simulink model "ospfb_2x_8192c_8i_16o"  (R2021a)
settings: Solver=VariableStepAuto  StartTime=0.0  StopTime=2304  RelTol=1e-3
undefined symbols (not defined in the file or in its folder): pfb_coeff_gen_calc
libraries: xbsIndex_r4 x23880, casper_library_misc x142, casper_library_multipliers x88, casper_library_delays x68
(part 7 of 158)

== system ospfb_2x_8192c_8i_16o/pfb_fir/pol1_in1_coeffs10 ==
  din : Inport
  sync : Inport  Port=2
  Concat : xbsIndex_r4/Concat  num_inputs=8  dbl_ovrd=off  block_type=concat
      settings: block_version=9.1.01
  Concat{1..8} (8 alike) : xbsIndex_r4/Concat  num_inputs=2  dbl_ovrd=off  block_type=concat
  Constant{,3,12,17} (4 alike) : xbsIndex_r4/Constant  const=0  gui_display_data_type=Fixed-point  arith_type=Unsigned  n_bits=1  bin_pt=0  preci_type=Single  exp_width=8  frac_width=24  explicit_period=on  period=1  equ=P=C  opselect=C  inp2=PCIN>>17  opr=+  inp1=P  carry=CIN  dbl_ovrd=off  block_type=constant
  Constant{1,4,13,18} (4 alike) : xbsIndex_r4/Constant  const=1  gui_display_data_type=Fixed-point  arith_type=Unsigned  n_bits=1  bin_pt=0  preci_type=Single  exp_width=8  frac_width=24  explicit_period=on  period=1  equ=P=C  opselect=C  inp2=PCIN>>17  opr=+  inp1=P  carry=CIN  dbl_ovrd=off  block_type=constant
  Constant{2,6,8,10,14,16,20,22} (8 alike) : xbsIndex_r4/Constant  const=0  gui_display_data_type=Fixed-point  arith_type=Signed (2's comp)  n_bits=18  bin_pt=17  preci_type=Single  exp_width=8  frac_width=24  explicit_period=on  period=1  equ=P=C  opselect=C  inp2=PCIN>>17  opr=+  inp1=P  carry=CIN  dbl_ovrd=off  block_type=constant
  Constant{5,7,9,11,15,19,21,23} (8 alike) : xbsIndex_r4/Constant  const=0  gui_display_data_type=Boolean  arith_type=Boolean  n_bits=18  bin_pt=17  preci_type=Single  exp_width=8  frac_width=24  explicit_period=on  period=1  equ=P=C  opselect=C  inp2=PCIN>>17  opr=+  inp1=P  carry=CIN  dbl_ovrd=off  block_type=constant
  Counter : xbsIndex_r4/Counter  cnt_type=Free Running  cnt_to=Inf  operation=Up  start_count=0  cnt_by_val=1  arith_type=Unsigned  n_bits=9  bin_pt=0  load_pin=off  rst=on  en=off  explicit_period=on  period=1  use_behavioral_HDL=off  implementation=Fabric  use_rpm=on  dbl_ovrd=off  block_type=counter
      settings: xl_area=[0, 0, 0, 0, 0, 0, 0]  block_version=9.1.01
  Delay : xbsIndex_r4/Delay  rst=off  infoeditControl=Selection of Reset will increase slice count due to use of real FFs and instead of SRLs  en=off  latency=bram_latency+fan_latency  reg_retiming=off  dbl_ovrd=off  block_type=delay
      settings: xl_area=[0, 0, 0, 0, 0, 0, 0]  block_version=9.1.01
  Delay1 : xbsIndex_r4/Delay  rst=off  infoeditControl=Selection of Reset will increase slice count due to use of real FFs and instead of SRLs  en=off  latency=bram_latency+fan_latency  reg_retiming=off  dbl_ovrd=off  block_type=delay
      settings: xl_area=[0, 0, 0, 0, 0, 0, 0]  block_version=9.1.01
  Reinterpret{1..8} (8 alike) : xbsIndex_r4/Reinterpret  force_arith_type=on  arith_type=Unsigned  force_bin_pt=on  bin_pt=0  block_type=reinterpret
      Reinterpret1 settings: infoedit="Changes signal type without altering the binary representation.   You can changed the signal between signed and unsigned, and relocate the binary point.<P><P>Hardware notes: In hardware this block costs nothing.<P><P>Example:  Suppose the input is 6 bits wide, signed, with 2 fractional bits, and the output is forced to unsigned with 0 fractional bits.  Then an input of -2.0 (1110.00 in binary 2's complement) becomes an output of 56 (111000 in binary)."  block_version=9.1.01
      Reinterpret2 settings: infoedit="Changes signal type without altering the binary representation.   You can changed the signal between signed and unsigned, and relocate the binary point.<P><P>Hardware notes: In hardware this block costs nothing.<P><P>Example:  Suppose the input is 6 bits wide, signed, with 2 fractional bits, and the output is forced to unsigned with 0 fractional bits.  Then an input of -2.0 (1110.00 in binary 2's complement) becomes an output of 56 (111000 in binary)."  block_version=9.1.01
  c0 : xbsIndex_r4/Dual Port RAM  depth=1024  initVector=[pfb_coeff_gen_calc(12, 8,'dpss',n_inputs, nput,1,1,false), pfb_coeff_gen_calc(12, 8,'dpss',n_inputs, nput,1,2,false)]  distributed_mem=Block RAM  init_a=0  init_b=0  rst_a=off  rst_b=off  en_a=off  en_b=off  latency=1  write_mode_A=Read After Write  write_mode_B=Read After Write  optimize=Area  dbl_ovrd=off  simulation_type=fast_sim  block_type=dpram
  c1 : xbsIndex_r4/Dual Port RAM  depth=1024  initVector=[pfb_coeff_gen_calc(12, 8,'dpss',n_inputs, nput,1,3,false), pfb_coeff_gen_calc(12, 8,'dpss',n_inputs, nput,1,4,false)]  distributed_mem=Block RAM  init_a=0  init_b=0  rst_a=off  rst_b=off  en_a=off  en_b=off  latency=1  write_mode_A=Read After Write  write_mode_B=Read After Write  optimize=Area  dbl_ovrd=off  simulation_type=fast_sim  block_type=dpram
  c2 : xbsIndex_r4/Dual Port RAM  depth=1024  initVector=[pfb_coeff_gen_calc(12, 8,'dpss',n_inputs, nput,1,5,false), pfb_coeff_gen_calc(12, 8,'dpss',n_inputs, nput,1,6,false)]  distributed_mem=Block RAM  init_a=0  init_b=0  rst_a=off  rst_b=off  en_a=off  en_b=off  latency=1  write_mode_A=Read After Write  write_mode_B=Read After Write  optimize=Area  dbl_ovrd=off  simulation_type=fast_sim  block_type=dpram
  c3 : xbsIndex_r4/Dual Port RAM  depth=1024  initVector=[pfb_coeff_gen_calc(12, 8,'dpss',n_inputs, nput,1,7,false), pfb_coeff_gen_calc(12, 8,'dpss',n_inputs, nput,1,8,false)]  distributed_mem=Block RAM  init_a=0  init_b=0  rst_a=off  rst_b=off  en_a=off  en_b=off  latency=1  write_mode_A=Read After Write  write_mode_B=Read After Write  optimize=Area  dbl_ovrd=off  simulation_type=fast_sim  block_type=dpram
  fan_delay{1..8} (8 alike) : xbsIndex_r4/Delay  rst=off  infoeditControl=Selection of Reset will increase slice count due to use of real FFs and instead of SRLs  en=off  latency=fan_latency  reg_retiming=off  dbl_ovrd=off  block_type=delay
      fan_delay1 settings: block_version=11.4
      fan_delay2 settings: block_version=11.4
  dout : Outport  VectorParamsAs1DForOutWhenUnconnected=off
  sync_out : Outport  Port=2  VectorParamsAs1DForOutWhenUnconnected=off
  coeff : Outport  Port=3  VectorParamsAs1DForOutWhenUnconnected=off
  wires:
  din/1 -> Delay1/1
  Delay1/1 -> dout/1
  sync/1 -> Counter/1, Delay/1
  Delay/1 -> sync_out/1
  Concat/1 -> coeff/1
  Counter/1 -> fan_delay1/1, fan_delay2/1, fan_delay3/1, fan_delay4/1, fan_delay5/1, fan_delay6/1, fan_delay7/1, fan_delay8/1
  fan_delay1/1 -> Concat1/2
  Reinterpret1/1 -> Concat/1
  fan_delay2/1 -> Concat2/2
  Reinterpret2/1 -> Concat/2
  Reinterpret3/1 -> Concat/3
  Reinterpret4/1 -> Concat/4
  Reinterpret5/1 -> Concat/5
  Reinterpret6/1 -> Concat/6
  Reinterpret7/1 -> Concat/7
  Reinterpret8/1 -> Concat/8
  Constant/1 -> Concat1/1
  Constant1/1 -> Concat2/1
  Concat1/1 -> c0/1
  Constant2/1 -> c0/2
  Constant5/1 -> c0/3
  Constant7/1 -> c0/6
  Constant6/1 -> c0/5
  Concat2/1 -> c0/4
  c0/2 -> Reinterpret2/1
  c0/1 -> Reinterpret1/1
  c1/2 -> Reinterpret4/1
  Concat4/1 -> c1/4
  Constant10/1 -> c1/5
  Constant11/1 -> c1/6
  Constant9/1 -> c1/3
  Constant8/1 -> c1/2
  Concat3/1 -> c1/1
  Constant4/1 -> Concat4/1
  Constant3/1 -> Concat3/1
  fan_delay4/1 -> Concat4/2
  fan_delay3/1 -> Concat3/2
  fan_delay7/1 -> Concat7/2
  fan_delay8/1 -> Concat8/2
  Constant17/1 -> Concat7/1
  Constant18/1 -> Concat8/1
  Concat7/1 -> c3/1
  Constant22/1 -> c3/2
  Constant23/1 -> c3/3
  Constant15/1 -> c3/6
  Constant14/1 -> c3/5
  Concat8/1 -> c3/4
  Concat6/1 -> c2/4
  Constant20/1 -> c2/5
  Constant21/1 -> c2/6
  Constant19/1 -> c2/3
  Constant16/1 -> c2/2
  Concat5/1 -> c2/1
  Constant13/1 -> Concat6/1
  Constant12/1 -> Concat5/1
  fan_delay6/1 -> Concat6/2
  fan_delay5/1 -> Concat5/2
  c1/1 -> Reinterpret3/1
  c2/1 -> Reinterpret5/1
  c2/2 -> Reinterpret6/1
  c3/1 -> Reinterpret7/1
  c3/2 -> Reinterpret8/1

== system ospfb_2x_8192c_8i_16o/pfb_fir/pol1_in1_coeffs11 ==
  din : Inport
  sync : Inport  Port=2
  Concat : xbsIndex_r4/Concat  num_inputs=8  dbl_ovrd=off  block_type=concat
      settings: block_version=9.1.01
  Concat{1..8} (8 alike) : xbsIndex_r4/Concat  num_inputs=2  dbl_ovrd=off  block_type=concat
  Constant{,3,12,17} (4 alike) : xbsIndex_r4/Constant  const=0  gui_display_data_type=Fixed-point  arith_type=Unsigned  n_bits=1  bin_pt=0  preci_type=Single  exp_width=8  frac_width=24  explicit_period=on  period=1  equ=P=C  opselect=C  inp2=PCIN>>17  opr=+  inp1=P  carry=CIN  dbl_ovrd=off  block_type=constant
  Constant{1,4,13,18} (4 alike) : xbsIndex_r4/Constant  const=1  gui_display_data_type=Fixed-point  arith_type=Unsigned  n_bits=1  bin_pt=0  preci_type=Single  exp_width=8  frac_width=24  explicit_period=on  period=1  equ=P=C  opselect=C  inp2=PCIN>>17  opr=+  inp1=P  carry=CIN  dbl_ovrd=off  block_type=constant
  Constant{2,6,8,10,14,16,20,22} (8 alike) : xbsIndex_r4/Constant  const=0  gui_display_data_type=Fixed-point  arith_type=Signed (2's comp)  n_bits=18  bin_pt=17  preci_type=Single  exp_width=8  frac_width=24  explicit_period=on  period=1  equ=P=C  opselect=C  inp2=PCIN>>17  opr=+  inp1=P  carry=CIN  dbl_ovrd=off  block_type=constant
  Constant{5,7,9,11,15,19,21,23} (8 alike) : xbsIndex_r4/Constant  const=0  gui_display_data_type=Boolean  arith_type=Boolean  n_bits=18  bin_pt=17  preci_type=Single  exp_width=8  frac_width=24  explicit_period=on  period=1  equ=P=C  opselect=C  inp2=PCIN>>17  opr=+  inp1=P  carry=CIN  dbl_ovrd=off  block_type=constant
  Counter : xbsIndex_r4/Counter  cnt_type=Free Running  cnt_to=Inf  operation=Up  start_count=0  cnt_by_val=1  arith_type=Unsigned  n_bits=9  bin_pt=0  load_pin=off  rst=on  en=off  explicit_period=on  period=1  use_behavioral_HDL=off  implementation=Fabric  use_rpm=on  dbl_ovrd=off  block_type=counter
      settings: xl_area=[0, 0, 0, 0, 0, 0, 0]  block_version=9.1.01
  Delay : xbsIndex_r4/Delay  rst=off  infoeditControl=Selection of Reset will increase slice count due to use of real FFs and instead of SRLs  en=off  latency=bram_latency+fan_latency  reg_retiming=off  dbl_ovrd=off  block_type=delay
      settings: xl_area=[0, 0, 0, 0, 0, 0, 0]  block_version=9.1.01
  Delay1 : xbsIndex_r4/Delay  rst=off  infoeditControl=Selection of Reset will increase slice count due to use of real FFs and instead of SRLs  en=off  latency=bram_latency+fan_latency  reg_retiming=off  dbl_ovrd=off  block_type=delay
      settings: xl_area=[0, 0, 0, 0, 0, 0, 0]  block_version=9.1.01
  Reinterpret{1..8} (8 alike) : xbsIndex_r4/Reinterpret  force_arith_type=on  arith_type=Unsigned  force_bin_pt=on  bin_pt=0  block_type=reinterpret
      Reinterpret1 settings: infoedit="Changes signal type without altering the binary representation.   You can changed the signal between signed and unsigned, and relocate the binary point.<P><P>Hardware notes: In hardware this block costs nothing.<P><P>Example:  Suppose the input is 6 bits wide, signed, with 2 fractional bits, and the output is forced to unsigned with 0 fractional bits.  Then an input of -2.0 (1110.00 in binary 2's complement) becomes an output of 56 (111000 in binary)."  block_version=9.1.01
      Reinterpret2 settings: infoedit="Changes signal type without altering the binary representation.   You can changed the signal between signed and unsigned, and relocate the binary point.<P><P>Hardware notes: In hardware this block costs nothing.<P><P>Example:  Suppose the input is 6 bits wide, signed, with 2 fractional bits, and the output is forced to unsigned with 0 fractional bits.  Then an input of -2.0 (1110.00 in binary 2's complement) becomes an output of 56 (111000 in binary)."  block_version=9.1.01
  c0 : xbsIndex_r4/Dual Port RAM  depth=1024  initVector=[pfb_coeff_gen_calc(12, 8,'dpss',n_inputs, nput,1,1,false), pfb_coeff_gen_calc(12, 8,'dpss',n_inputs, nput,1,2,false)]  distributed_mem=Block RAM  init_a=0  init_b=0  rst_a=off  rst_b=off  en_a=off  en_b=off  latency=1  write_mode_A=Read After Write  write_mode_B=Read After Write  optimize=Area  dbl_ovrd=off  simulation_type=fast_sim  block_type=dpram
  c1 : xbsIndex_r4/Dual Port RAM  depth=1024  initVector=[pfb_coeff_gen_calc(12, 8,'dpss',n_inputs, nput,1,3,false), pfb_coeff_gen_calc(12, 8,'dpss',n_inputs, nput,1,4,false)]  distributed_mem=Block RAM  init_a=0  init_b=0  rst_a=off  rst_b=off  en_a=off  en_b=off  latency=1  write_mode_A=Read After Write  write_mode_B=Read After Write  optimize=Area  dbl_ovrd=off  simulation_type=fast_sim  block_type=dpram
  c2 : xbsIndex_r4/Dual Port RAM  depth=1024  initVector=[pfb_coeff_gen_calc(12, 8,'dpss',n_inputs, nput,1,5,false), pfb_coeff_gen_calc(12, 8,'dpss',n_inputs, nput,1,6,false)]  distributed_mem=Block RAM  init_a=0  init_b=0  rst_a=off  rst_b=off  en_a=off  en_b=off  latency=1  write_mode_A=Read After Write  write_mode_B=Read After Write  optimize=Area  dbl_ovrd=off  simulation_type=fast_sim  block_type=dpram
  c3 : xbsIndex_r4/Dual Port RAM  depth=1024  initVector=[pfb_coeff_gen_calc(12, 8,'dpss',n_inputs, nput,1,7,false), pfb_coeff_gen_calc(12, 8,'dpss',n_inputs, nput,1,8,false)]  distributed_mem=Block RAM  init_a=0  init_b=0  rst_a=off  rst_b=off  en_a=off  en_b=off  latency=1  write_mode_A=Read After Write  write_mode_B=Read After Write  optimize=Area  dbl_ovrd=off  simulation_type=fast_sim  block_type=dpram
  fan_delay{1..8} (8 alike) : xbsIndex_r4/Delay  rst=off  infoeditControl=Selection of Reset will increase slice count due to use of real FFs and instead of SRLs  en=off  latency=fan_latency  reg_retiming=off  dbl_ovrd=off  block_type=delay
      fan_delay1 settings: block_version=11.4
      fan_delay2 settings: block_version=11.4
  dout : Outport  VectorParamsAs1DForOutWhenUnconnected=off
  sync_out : Outport  Port=2  VectorParamsAs1DForOutWhenUnconnected=off
  coeff : Outport  Port=3  VectorParamsAs1DForOutWhenUnconnected=off
  wires:
  c3/2 -> Reinterpret8/1
  c3/1 -> Reinterpret7/1
  c2/2 -> Reinterpret6/1
  c2/1 -> Reinterpret5/1
  c1/1 -> Reinterpret3/1
  fan_delay5/1 -> Concat5/2
  fan_delay6/1 -> Concat6/2
  Constant12/1 -> Concat5/1
  Constant13/1 -> Concat6/1
  Concat5/1 -> c2/1
  Constant16/1 -> c2/2
  Constant19/1 -> c2/3
  Constant21/1 -> c2/6
  Constant20/1 -> c2/5
  Concat6/1 -> c2/4
  Concat8/1 -> c3/4
  Constant14/1 -> c3/5
  Constant15/1 -> c3/6
  Constant23/1 -> c3/3
  Constant22/1 -> c3/2
  Concat7/1 -> c3/1
  Constant18/1 -> Concat8/1
  Constant17/1 -> Concat7/1
  fan_delay8/1 -> Concat8/2
  fan_delay7/1 -> Concat7/2
  fan_delay3/1 -> Concat3/2
  fan_delay4/1 -> Concat4/2
  Constant3/1 -> Concat3/1
  Constant4/1 -> Concat4/1
  Concat3/1 -> c1/1
  Constant8/1 -> c1/2
  Constant9/1 -> c1/3
  Constant11/1 -> c1/6
  Constant10/1 -> c1/5
  Concat4/1 -> c1/4
  c1/2 -> Reinterpret4/1
  c0/1 -> Reinterpret1/1
  c0/2 -> Reinterpret2/1
  Concat2/1 -> c0/4
  Constant6/1 -> c0/5
  Constant7/1 -> c0/6
  Constant5/1 -> c0/3
  Constant2/1 -> c0/2
  Concat1/1 -> c0/1
  Constant1/1 -> Concat2/1
  Constant/1 -> Concat1/1
  Reinterpret8/1 -> Concat/8
  Reinterpret7/1 -> Concat/7
  Reinterpret6/1 -> Concat/6
  Reinterpret5/1 -> Concat/5
  Reinterpret4/1 -> Concat/4
  Reinterpret3/1 -> Concat/3
  Reinterpret2/1 -> Concat/2
  fan_delay2/1 -> Concat2/2
  Reinterpret1/1 -> Concat/1
  fan_delay1/1 -> Concat1/2
  Counter/1 -> fan_delay8/1, fan_delay7/1, fan_delay6/1, fan_delay5/1, fan_delay4/1, fan_delay3/1, fan_delay2/1, fan_delay1/1
  Concat/1 -> coeff/1
  Delay/1 -> sync_out/1
  sync/1 -> Delay/1, Counter/1
  Delay1/1 -> dout/1
  din/1 -> Delay1/1

== system ospfb_2x_8192c_8i_16o/pfb_fir/pol1_in1_coeffs12 ==
  din : Inport
  sync : Inport  Port=2
  Concat : xbsIndex_r4/Concat  num_inputs=8  dbl_ovrd=off  block_type=concat
      settings: block_version=9.1.01
  Concat{1..8} (8 alike) : xbsIndex_r4/Concat  num_inputs=2  dbl_ovrd=off  block_type=concat
  Constant{,3,12,17} (4 alike) : xbsIndex_r4/Constant  const=0  gui_display_data_type=Fixed-point  arith_type=Unsigned  n_bits=1  bin_pt=0  preci_type=Single  exp_width=8  frac_width=24  explicit_period=on  period=1  equ=P=C  opselect=C  inp2=PCIN>>17  opr=+  inp1=P  carry=CIN  dbl_ovrd=off  block_type=constant
  Constant{1,4,13,18} (4 alike) : xbsIndex_r4/Constant  const=1  gui_display_data_type=Fixed-point  arith_type=Unsigned  n_bits=1  bin_pt=0  preci_type=Single  exp_width=8  frac_width=24  explicit_period=on  period=1  equ=P=C  opselect=C  inp2=PCIN>>17  opr=+  inp1=P  carry=CIN  dbl_ovrd=off  block_type=constant
  Constant{2,6,8,10,14,16,20,22} (8 alike) : xbsIndex_r4/Constant  const=0  gui_display_data_type=Fixed-point  arith_type=Signed (2's comp)  n_bits=18  bin_pt=17  preci_type=Single  exp_width=8  frac_width=24  explicit_period=on  period=1  equ=P=C  opselect=C  inp2=PCIN>>17  opr=+  inp1=P  carry=CIN  dbl_ovrd=off  block_type=constant
  Constant{5,7,9,11,15,19,21,23} (8 alike) : xbsIndex_r4/Constant  const=0  gui_display_data_type=Boolean  arith_type=Boolean  n_bits=18  bin_pt=17  preci_type=Single  exp_width=8  frac_width=24  explicit_period=on  period=1  equ=P=C  opselect=C  inp2=PCIN>>17  opr=+  inp1=P  carry=CIN  dbl_ovrd=off  block_type=constant
  Counter : xbsIndex_r4/Counter  cnt_type=Free Running  cnt_to=Inf  operation=Up  start_count=0  cnt_by_val=1  arith_type=Unsigned  n_bits=9  bin_pt=0  load_pin=off  rst=on  en=off  explicit_period=on  period=1  use_behavioral_HDL=off  implementation=Fabric  use_rpm=on  dbl_ovrd=off  block_type=counter
      settings: xl_area=[0, 0, 0, 0, 0, 0, 0]  block_version=9.1.01
  Delay : xbsIndex_r4/Delay  rst=off  infoeditControl=Selection of Reset will increase slice count due to use of real FFs and instead of SRLs  en=off  latency=bram_latency+fan_latency  reg_retiming=off  dbl_ovrd=off  block_type=delay
      settings: xl_area=[0, 0, 0, 0, 0, 0, 0]  block_version=9.1.01
  Delay1 : xbsIndex_r4/Delay  rst=off  infoeditControl=Selection of Reset will increase slice count due to use of real FFs and instead of SRLs  en=off  latency=bram_latency+fan_latency  reg_retiming=off  dbl_ovrd=off  block_type=delay
      settings: xl_area=[0, 0, 0, 0, 0, 0, 0]  block_version=9.1.01
  Reinterpret{1..8} (8 alike) : xbsIndex_r4/Reinterpret  force_arith_type=on  arith_type=Unsigned  force_bin_pt=on  bin_pt=0  block_type=reinterpret
      Reinterpret1 settings: infoedit="Changes signal type without altering the binary representation.   You can changed the signal between signed and unsigned, and relocate the binary point.<P><P>Hardware notes: In hardware this block costs nothing.<P><P>Example:  Suppose the input is 6 bits wide, signed, with 2 fractional bits, and the output is forced to unsigned with 0 fractional bits.  Then an input of -2.0 (1110.00 in binary 2's complement) becomes an output of 56 (111000 in binary)."  block_version=9.1.01
      Reinterpret2 settings: infoedit="Changes signal type without altering the binary representation.   You can changed the signal between signed and unsigned, and relocate the binary point.<P><P>Hardware notes: In hardware this block costs nothing.<P><P>Example:  Suppose the input is 6 bits wide, signed, with 2 fractional bits, and the output is forced to unsigned with 0 fractional bits.  Then an input of -2.0 (1110.00 in binary 2's complement) becomes an output of 56 (111000 in binary)."  block_version=9.1.01
  c0 : xbsIndex_r4/Dual Port RAM  depth=1024  initVector=[pfb_coeff_gen_calc(12, 8,'dpss',n_inputs, nput,1,1,false), pfb_coeff_gen_calc(12, 8,'dpss',n_inputs, nput,1,2,false)]  distributed_mem=Block RAM  init_a=0  init_b=0  rst_a=off  rst_b=off  en_a=off  en_b=off  latency=1  write_mode_A=Read After Write  write_mode_B=Read After Write  optimize=Area  dbl_ovrd=off  simulation_type=fast_sim  block_type=dpram
  c1 : xbsIndex_r4/Dual Port RAM  depth=1024  initVector=[pfb_coeff_gen_calc(12, 8,'dpss',n_inputs, nput,1,3,false), pfb_coeff_gen_calc(12, 8,'dpss',n_inputs, nput,1,4,false)]  distributed_mem=Block RAM  init_a=0  init_b=0  rst_a=off  rst_b=off  en_a=off  en_b=off  latency=1  write_mode_A=Read After Write  write_mode_B=Read After Write  optimize=Area  dbl_ovrd=off  simulation_type=fast_sim  block_type=dpram
  c2 : xbsIndex_r4/Dual Port RAM  depth=1024  initVector=[pfb_coeff_gen_calc(12, 8,'dpss',n_inputs, nput,1,5,false), pfb_coeff_gen_calc(12, 8,'dpss',n_inputs, nput,1,6,false)]  distributed_mem=Block RAM  init_a=0  init_b=0  rst_a=off  rst_b=off  en_a=off  en_b=off  latency=1  write_mode_A=Read After Write  write_mode_B=Read After Write  optimize=Area  dbl_ovrd=off  simulation_type=fast_sim  block_type=dpram
  c3 : xbsIndex_r4/Dual Port RAM  depth=1024  initVector=[pfb_coeff_gen_calc(12, 8,'dpss',n_inputs, nput,1,7,false), pfb_coeff_gen_calc(12, 8,'dpss',n_inputs, nput,1,8,false)]  distributed_mem=Block RAM  init_a=0  init_b=0  rst_a=off  rst_b=off  en_a=off  en_b=off  latency=1  write_mode_A=Read After Write  write_mode_B=Read After Write  optimize=Area  dbl_ovrd=off  simulation_type=fast_sim  block_type=dpram
  fan_delay{1..8} (8 alike) : xbsIndex_r4/Delay  rst=off  infoeditControl=Selection of Reset will increase slice count due to use of real FFs and instead of SRLs  en=off  latency=fan_latency  reg_retiming=off  dbl_ovrd=off  block_type=delay
      fan_delay1 settings: block_version=11.4
      fan_delay2 settings: block_version=11.4
  dout : Outport  VectorParamsAs1DForOutWhenUnconnected=off
  sync_out : Outport  Port=2  VectorParamsAs1DForOutWhenUnconnected=off
  coeff : Outport  Port=3  VectorParamsAs1DForOutWhenUnconnected=off
  wires:
  din/1 -> Delay1/1
  Delay1/1 -> dout/1
  sync/1 -> Counter/1, Delay/1
  Delay/1 -> sync_out/1
  Concat/1 -> coeff/1
  Counter/1 -> fan_delay1/1, fan_delay2/1, fan_delay3/1, fan_delay4/1, fan_delay5/1, fan_delay6/1, fan_delay7/1, fan_delay8/1
  fan_delay1/1 -> Concat1/2
  Reinterpret1/1 -> Concat/1
  fan_delay2/1 -> Concat2/2
  Reinterpret2/1 -> Concat/2
  Reinterpret3/1 -> Concat/3
  Reinterpret4/1 -> Concat/4
  Reinterpret5/1 -> Concat/5
  Reinterpret6/1 -> Concat/6
  Reinterpret7/1 -> Concat/7
  Reinterpret8/1 -> Concat/8
  Constant/1 -> Concat1/1
  Constant1/1 -> Concat2/1
  Concat1/1 -> c0/1
  Constant2/1 -> c0/2
  Constant5/1 -> c0/3
  Constant7/1 -> c0/6
  Constant6/1 -> c0/5
  Concat2/1 -> c0/4
  c0/2 -> Reinterpret2/1
  c0/1 -> Reinterpret1/1
  c1/2 -> Reinterpret4/1
  Concat4/1 -> c1/4
  Constant10/1 -> c1/5
  Constant11/1 -> c1/6
  Constant9/1 -> c1/3
  Constant8/1 -> c1/2
  Concat3/1 -> c1/1
  Constant4/1 -> Concat4/1
  Constant3/1 -> Concat3/1
  fan_delay4/1 -> Concat4/2
  fan_delay3/1 -> Concat3/2
  fan_delay7/1 -> Concat7/2
  fan_delay8/1 -> Concat8/2
  Constant17/1 -> Concat7/1
  Constant18/1 -> Concat8/1
  Concat7/1 -> c3/1
  Constant22/1 -> c3/2
  Constant23/1 -> c3/3
  Constant15/1 -> c3/6
  Constant14/1 -> c3/5
  Concat8/1 -> c3/4
  Concat6/1 -> c2/4
  Constant20/1 -> c2/5
  Constant21/1 -> c2/6
  Constant19/1 -> c2/3
  Constant16/1 -> c2/2
  Concat5/1 -> c2/1
  Constant13/1 -> Concat6/1
  Constant12/1 -> Concat5/1
  fan_delay6/1 -> Concat6/2
  fan_delay5/1 -> Concat5/2
  c1/1 -> Reinterpret3/1
  c2/1 -> Reinterpret5/1
  c2/2 -> Reinterpret6/1
  c3/1 -> Reinterpret7/1
  c3/2 -> Reinterpret8/1

== system ospfb_2x_8192c_8i_16o/pfb_fir/pol1_in1_coeffs13 ==
  din : Inport
  sync : Inport  Port=2
  Concat : xbsIndex_r4/Concat  num_inputs=8  dbl_ovrd=off  block_type=concat
      settings: block_version=9.1.01
  Concat{1..8} (8 alike) : xbsIndex_r4/Concat  num_inputs=2  dbl_ovrd=off  block_type=concat
  Constant{,3,12,17} (4 alike) : xbsIndex_r4/Constant  const=0  gui_display_data_type=Fixed-point  arith_type=Unsigned  n_bits=1  bin_pt=0  preci_type=Single  exp_width=8  frac_width=24  explicit_period=on  period=1  equ=P=C  opselect=C  inp2=PCIN>>17  opr=+  inp1=P  carry=CIN  dbl_ovrd=off  block_type=constant
  Constant{1,4,13,18} (4 alike) : xbsIndex_r4/Constant  const=1  gui_display_data_type=Fixed-point  arith_type=Unsigned  n_bits=1  bin_pt=0  preci_type=Single  exp_width=8  frac_width=24  explicit_period=on  period=1  equ=P=C  opselect=C  inp2=PCIN>>17  opr=+  inp1=P  carry=CIN  dbl_ovrd=off  block_type=constant
  Constant{2,6,8,10,14,16,20,22} (8 alike) : xbsIndex_r4/Constant  const=0  gui_display_data_type=Fixed-point  arith_type=Signed (2's comp)  n_bits=18  bin_pt=17  preci_type=Single  exp_width=8  frac_width=24  explicit_period=on  period=1  equ=P=C  opselect=C  inp2=PCIN>>17  opr=+  inp1=P  carry=CIN  dbl_ovrd=off  block_type=constant
  Constant{5,7,9,11,15,19,21,23} (8 alike) : xbsIndex_r4/Constant  const=0  gui_display_data_type=Boolean  arith_type=Boolean  n_bits=18  bin_pt=17  preci_type=Single  exp_width=8  frac_width=24  explicit_period=on  period=1  equ=P=C  opselect=C  inp2=PCIN>>17  opr=+  inp1=P  carry=CIN  dbl_ovrd=off  block_type=constant
  Counter : xbsIndex_r4/Counter  cnt_type=Free Running  cnt_to=Inf  operation=Up  start_count=0  cnt_by_val=1  arith_type=Unsigned  n_bits=9  bin_pt=0  load_pin=off  rst=on  en=off  explicit_period=on  period=1  use_behavioral_HDL=off  implementation=Fabric  use_rpm=on  dbl_ovrd=off  block_type=counter
      settings: xl_area=[0, 0, 0, 0, 0, 0, 0]  block_version=9.1.01
  Delay : xbsIndex_r4/Delay  rst=off  infoeditControl=Selection of Reset will increase slice count due to use of real FFs and instead of SRLs  en=off  latency=bram_latency+fan_latency  reg_retiming=off  dbl_ovrd=off  block_type=delay
      settings: xl_area=[0, 0, 0, 0, 0, 0, 0]  block_version=9.1.01
  Delay1 : xbsIndex_r4/Delay  rst=off  infoeditControl=Selection of Reset will increase slice count due to use of real FFs and instead of SRLs  en=off  latency=bram_latency+fan_latency  reg_retiming=off  dbl_ovrd=off  block_type=delay
      settings: xl_area=[0, 0, 0, 0, 0, 0, 0]  block_version=9.1.01
  Reinterpret{1..8} (8 alike) : xbsIndex_r4/Reinterpret  force_arith_type=on  arith_type=Unsigned  force_bin_pt=on  bin_pt=0  block_type=reinterpret
      Reinterpret1 settings: infoedit="Changes signal type without altering the binary representation.   You can changed the signal between signed and unsigned, and relocate the binary point.<P><P>Hardware notes: In hardware this block costs nothing.<P><P>Example:  Suppose the input is 6 bits wide, signed, with 2 fractional bits, and the output is forced to unsigned with 0 fractional bits.  Then an input of -2.0 (1110.00 in binary 2's complement) becomes an output of 56 (111000 in binary)."  block_version=9.1.01
      Reinterpret2 settings: infoedit="Changes signal type without altering the binary representation.   You can changed the signal between signed and unsigned, and relocate the binary point.<P><P>Hardware notes: In hardware this block costs nothing.<P><P>Example:  Suppose the input is 6 bits wide, signed, with 2 fractional bits, and the output is forced to unsigned with 0 fractional bits.  Then an input of -2.0 (1110.00 in binary 2's complement) becomes an output of 56 (111000 in binary)."  block_version=9.1.01
  c0 : xbsIndex_r4/Dual Port RAM  depth=1024  initVector=[pfb_coeff_gen_calc(12, 8,'dpss',n_inputs, nput,1,1,false), pfb_coeff_gen_calc(12, 8,'dpss',n_inputs, nput,1,2,false)]  distributed_mem=Block RAM  init_a=0  init_b=0  rst_a=off  rst_b=off  en_a=off  en_b=off  latency=1  write_mode_A=Read After Write  write_mode_B=Read After Write  optimize=Area  dbl_ovrd=off  simulation_type=fast_sim  block_type=dpram
  c1 : xbsIndex_r4/Dual Port RAM  depth=1024  initVector=[pfb_coeff_gen_calc(12, 8,'dpss',n_inputs, nput,1,3,false), pfb_coeff_gen_calc(12, 8,'dpss',n_inputs, nput,1,4,false)]  distributed_mem=Block RAM  init_a=0  init_b=0  rst_a=off  rst_b=off  en_a=off  en_b=off  latency=1  write_mode_A=Read After Write  write_mode_B=Read After Write  optimize=Area  dbl_ovrd=off  simulation_type=fast_sim  block_type=dpram
  c2 : xbsIndex_r4/Dual Port RAM  depth=1024  initVector=[pfb_coeff_gen_calc(12, 8,'dpss',n_inputs, nput,1,5,false), pfb_coeff_gen_calc(12, 8,'dpss',n_inputs, nput,1,6,false)]  distributed_mem=Block RAM  init_a=0  init_b=0  rst_a=off  rst_b=off  en_a=off  en_b=off  latency=1  write_mode_A=Read After Write  write_mode_B=Read After Write  optimize=Area  dbl_ovrd=off  simulation_type=fast_sim  block_type=dpram
  c3 : xbsIndex_r4/Dual Port RAM  depth=1024  initVector=[pfb_coeff_gen_calc(12, 8,'dpss',n_inputs, nput,1,7,false), pfb_coeff_gen_calc(12, 8,'dpss',n_inputs, nput,1,8,false)]  distributed_mem=Block RAM  init_a=0  init_b=0  rst_a=off  rst_b=off  en_a=off  en_b=off  latency=1  write_mode_A=Read After Write  write_mode_B=Read After Write  optimize=Area  dbl_ovrd=off  simulation_type=fast_sim  block_type=dpram
  fan_delay{1..8} (8 alike) : xbsIndex_r4/Delay  rst=off  infoeditControl=Selection of Reset will increase slice count due to use of real FFs and instead of SRLs  en=off  latency=fan_latency  reg_retiming=off  dbl_ovrd=off  block_type=delay
      fan_delay1 settings: block_version=11.4
      fan_delay2 settings: block_version=11.4
  dout : Outport  VectorParamsAs1DForOutWhenUnconnected=off
  sync_out : Outport  Port=2  VectorParamsAs1DForOutWhenUnconnected=off
  coeff : Outport  Port=3  VectorParamsAs1DForOutWhenUnconnected=off
  wires:
  c3/2 -> Reinterpret8/1
  c3/1 -> Reinterpret7/1
  c2/2 -> Reinterpret6/1
  c2/1 -> Reinterpret5/1
  c1/1 -> Reinterpret3/1
  fan_delay5/1 -> Concat5/2
  fan_delay6/1 -> Concat6/2
  Constant12/1 -> Concat5/1
  Constant13/1 -> Concat6/1
  Concat5/1 -> c2/1
  Constant16/1 -> c2/2
  Constant19/1 -> c2/3
  Constant21/1 -> c2/6
  Constant20/1 -> c2/5
  Concat6/1 -> c2/4
  Concat8/1 -> c3/4
  Constant14/1 -> c3/5
  Constant15/1 -> c3/6
  Constant23/1 -> c3/3
  Constant22/1 -> c3/2
  Concat7/1 -> c3/1
  Constant18/1 -> Concat8/1
  Constant17/1 -> Concat7/1
  fan_delay8/1 -> Concat8/2
  fan_delay7/1 -> Concat7/2
  fan_delay3/1 -> Concat3/2
  fan_delay4/1 -> Concat4/2
  Constant3/1 -> Concat3/1
  Constant4/1 -> Concat4/1
  Concat3/1 -> c1/1
  Constant8/1 -> c1/2
  Constant9/1 -> c1/3
  Constant11/1 -> c1/6
  Constant10/1 -> c1/5
  Concat4/1 -> c1/4
  c1/2 -> Reinterpret4/1
  c0/1 -> Reinterpret1/1
  c0/2 -> Reinterpret2/1
  Concat2/1 -> c0/4
  Constant6/1 -> c0/5
  Constant7/1 -> c0/6
  Constant5/1 -> c0/3
  Constant2/1 -> c0/2
  Concat1/1 -> c0/1
  Constant1/1 -> Concat2/1
  Constant/1 -> Concat1/1
  Reinterpret8/1 -> Concat/8
  Reinterpret7/1 -> Concat/7
  Reinterpret6/1 -> Concat/6
  Reinterpret5/1 -> Concat/5
  Reinterpret4/1 -> Concat/4
  Reinterpret3/1 -> Concat/3
  Reinterpret2/1 -> Concat/2
  fan_delay2/1 -> Concat2/2
  Reinterpret1/1 -> Concat/1
  fan_delay1/1 -> Concat1/2
  Counter/1 -> fan_delay8/1, fan_delay7/1, fan_delay6/1, fan_delay5/1, fan_delay4/1, fan_delay3/1, fan_delay2/1, fan_delay1/1
  Concat/1 -> coeff/1
  Delay/1 -> sync_out/1
  sync/1 -> Delay/1, Counter/1
  Delay1/1 -> dout/1
  din/1 -> Delay1/1

== system ospfb_2x_8192c_8i_16o/pfb_fir/pol1_in1_coeffs14 ==
  din : Inport
  sync : Inport  Port=2
  Concat : xbsIndex_r4/Concat  num_inputs=8  dbl_ovrd=off  block_type=concat
      settings: block_version=9.1.01
  Concat{1..8} (8 alike) : xbsIndex_r4/Concat  num_inputs=2  dbl_ovrd=off  block_type=concat
  Constant{,3,12,17} (4 alike) : xbsIndex_r4/Constant  const=0  gui_display_data_type=Fixed-point  arith_type=Unsigned  n_bits=1  bin_pt=0  preci_type=Single  exp_width=8  frac_width=24  explicit_period=on  period=1  equ=P=C  opselect=C  inp2=PCIN>>17  opr=+  inp1=P  carry=CIN  dbl_ovrd=off  block_type=constant
  Constant{1,4,13,18} (4 alike) : xbsIndex_r4/Constant  const=1  gui_display_data_type=Fixed-point  arith_type=Unsigned  n_bits=1  bin_pt=0  preci_type=Single  exp_width=8  frac_width=24  explicit_period=on  period=1  equ=P=C  opselect=C  inp2=PCIN>>17  opr=+  inp1=P  carry=CIN  dbl_ovrd=off  block_type=constant
  Constant{2,6,8,10,14,16,20,22} (8 alike) : xbsIndex_r4/Constant  const=0  gui_display_data_type=Fixed-point  arith_type=Signed (2's comp)  n_bits=18  bin_pt=17  preci_type=Single  exp_width=8  frac_width=24  explicit_period=on  period=1  equ=P=C  opselect=C  inp2=PCIN>>17  opr=+  inp1=P  carry=CIN  dbl_ovrd=off  block_type=constant
  Constant{5,7,9,11,15,19,21,23} (8 alike) : xbsIndex_r4/Constant  const=0  gui_display_data_type=Boolean  arith_type=Boolean  n_bits=18  bin_pt=17  preci_type=Single  exp_width=8  frac_width=24  explicit_period=on  period=1  equ=P=C  opselect=C  inp2=PCIN>>17  opr=+  inp1=P  carry=CIN  dbl_ovrd=off  block_type=constant
  Counter : xbsIndex_r4/Counter  cnt_type=Free Running  cnt_to=Inf  operation=Up  start_count=0  cnt_by_val=1  arith_type=Unsigned  n_bits=9  bin_pt=0  load_pin=off  rst=on  en=off  explicit_period=on  period=1  use_behavioral_HDL=off  implementation=Fabric  use_rpm=on  dbl_ovrd=off  block_type=counter
      settings: xl_area=[0, 0, 0, 0, 0, 0, 0]  block_version=9.1.01
  Delay : xbsIndex_r4/Delay  rst=off  infoeditControl=Selection of Reset will increase slice count due to use of real FFs and instead of SRLs  en=off  latency=bram_latency+fan_latency  reg_retiming=off  dbl_ovrd=off  block_type=delay
      settings: xl_area=[0, 0, 0, 0, 0, 0, 0]  block_version=9.1.01
  Delay1 : xbsIndex_r4/Delay  rst=off  infoeditControl=Selection of Reset will increase slice count due to use of real FFs and instead of SRLs  en=off  latency=bram_latency+fan_latency  reg_retiming=off  dbl_ovrd=off  block_type=delay
      settings: xl_area=[0, 0, 0, 0, 0, 0, 0]  block_version=9.1.01
  Reinterpret{1..8} (8 alike) : xbsIndex_r4/Reinterpret  force_arith_type=on  arith_type=Unsigned  force_bin_pt=on  bin_pt=0  block_type=reinterpret
      Reinterpret1 settings: infoedit="Changes signal type without altering the binary representation.   You can changed the signal between signed and unsigned, and relocate the binary point.<P><P>Hardware notes: In hardware this block costs nothing.<P><P>Example:  Suppose the input is 6 bits wide, signed, with 2 fractional bits, and the output is forced to unsigned with 0 fractional bits.  Then an input of -2.0 (1110.00 in binary 2's complement) becomes an output of 56 (111000 in binary)."  block_version=9.1.01
      Reinterpret2 settings: infoedit="Changes signal type without altering the binary representation.   You can changed the signal between signed and unsigned, and relocate the binary point.<P><P>Hardware notes: In hardware this block costs nothing.<P><P>Example:  Suppose the input is 6 bits wide, signed, with 2 fractional bits, and the output is forced to unsigned with 0 fractional bits.  Then an input of -2.0 (1110.00 in binary 2's complement) becomes an output of 56 (111000 in binary)."  block_version=9.1.01
  c0 : xbsIndex_r4/Dual Port RAM  depth=1024  initVector=[pfb_coeff_gen_calc(12, 8,'dpss',n_inputs, nput,1,1,false), pfb_coeff_gen_calc(12, 8,'dpss',n_inputs, nput,1,2,false)]  distributed_mem=Block RAM  init_a=0  init_b=0  rst_a=off  rst_b=off  en_a=off  en_b=off  latency=1  write_mode_A=Read After Write  write_mode_B=Read After Write  optimize=Area  dbl_ovrd=off  simulation_type=fast_sim  block_type=dpram
  c1 : xbsIndex_r4/Dual Port RAM  depth=1024  initVector=[pfb_coeff_gen_calc(12, 8,'dpss',n_inputs, nput,1,3,false), pfb_coeff_gen_calc(12, 8,'dpss',n_inputs, nput,1,4,false)]  distributed_mem=Block RAM  init_a=0  init_b=0  rst_a=off  rst_b=off  en_a=off  en_b=off  latency=1  write_mode_A=Read After Write  write_mode_B=Read After Write  optimize=Area  dbl_ovrd=off  simulation_type=fast_sim  block_type=dpram
  c2 : xbsIndex_r4/Dual Port RAM  depth=1024  initVector=[pfb_coeff_gen_calc(12, 8,'dpss',n_inputs, nput,1,5,false), pfb_coeff_gen_calc(12, 8,'dpss',n_inputs, nput,1,6,false)]  distributed_mem=Block RAM  init_a=0  init_b=0  rst_a=off  rst_b=off  en_a=off  en_b=off  latency=1  write_mode_A=Read After Write  write_mode_B=Read After Write  optimize=Area  dbl_ovrd=off  simulation_type=fast_sim  block_type=dpram
  c3 : xbsIndex_r4/Dual Port RAM  depth=1024  initVector=[pfb_coeff_gen_calc(12, 8,'dpss',n_inputs, nput,1,7,false), pfb_coeff_gen_calc(12, 8,'dpss',n_inputs, nput,1,8,false)]  distributed_mem=Block RAM  init_a=0  init_b=0  rst_a=off  rst_b=off  en_a=off  en_b=off  latency=1  write_mode_A=Read After Write  write_mode_B=Read After Write  optimize=Area  dbl_ovrd=off  simulation_type=fast_sim  block_type=dpram
  fan_delay{1..8} (8 alike) : xbsIndex_r4/Delay  rst=off  infoeditControl=Selection of Reset will increase slice count due to use of real FFs and instead of SRLs  en=off  latency=fan_latency  reg_retiming=off  dbl_ovrd=off  block_type=delay
      fan_delay1 settings: block_version=11.4
      fan_delay2 settings: block_version=11.4
  dout : Outport  VectorParamsAs1DForOutWhenUnconnected=off
  sync_out : Outport  Port=2  VectorParamsAs1DForOutWhenUnconnected=off
  coeff : Outport  Port=3  VectorParamsAs1DForOutWhenUnconnected=off
  wires:
  din/1 -> Delay1/1
  Delay1/1 -> dout/1
  sync/1 -> Counter/1, Delay/1
  Delay/1 -> sync_out/1
  Concat/1 -> coeff/1
  Counter/1 -> fan_delay1/1, fan_delay2/1, fan_delay3/1, fan_delay4/1, fan_delay5/1, fan_delay6/1, fan_delay7/1, fan_delay8/1
  fan_delay1/1 -> Concat1/2
  Reinterpret1/1 -> Concat/1
  fan_delay2/1 -> Concat2/2
  Reinterpret2/1 -> Concat/2
  Reinterpret3/1 -> Concat/3
  Reinterpret4/1 -> Concat/4
  Reinterpret5/1 -> Concat/5
  Reinterpret6/1 -> Concat/6
  Reinterpret7/1 -> Concat/7
  Reinterpret8/1 -> Concat/8
  Constant/1 -> Concat1/1
  Constant1/1 -> Concat2/1
  Concat1/1 -> c0/1
  Constant2/1 -> c0/2
  Constant5/1 -> c0/3
  Constant7/1 -> c0/6
  Constant6/1 -> c0/5
  Concat2/1 -> c0/4
  c0/2 -> Reinterpret2/1
  c0/1 -> Reinterpret1/1
  c1/2 -> Reinterpret4/1
  Concat4/1 -> c1/4
  Constant10/1 -> c1/5
  Constant11/1 -> c1/6
  Constant9/1 -> c1/3
  Constant8/1 -> c1/2
  Concat3/1 -> c1/1
  Constant4/1 -> Concat4/1
  Constant3/1 -> Concat3/1
  fan_delay4/1 -> Concat4/2
  fan_delay3/1 -> Concat3/2
  fan_delay7/1 -> Concat7/2
  fan_delay8/1 -> Concat8/2
  Constant17/1 -> Concat7/1
  Constant18/1 -> Concat8/1
  Concat7/1 -> c3/1
  Constant22/1 -> c3/2
  Constant23/1 -> c3/3
  Constant15/1 -> c3/6
  Constant14/1 -> c3/5
  Concat8/1 -> c3/4
  Concat6/1 -> c2/4
  Constant20/1 -> c2/5
  Constant21/1 -> c2/6
  Constant19/1 -> c2/3
  Constant16/1 -> c2/2
  Concat5/1 -> c2/1
  Constant13/1 -> Concat6/1
  Constant12/1 -> Concat5/1
  fan_delay6/1 -> Concat6/2
  fan_delay5/1 -> Concat5/2
  c1/1 -> Reinterpret3/1
  c2/1 -> Reinterpret5/1
  c2/2 -> Reinterpret6/1
  c3/1 -> Reinterpret7/1
  c3/2 -> Reinterpret8/1

== system ospfb_2x_8192c_8i_16o/pfb_fir/pol1_in1_coeffs15 ==
  din : Inport
  sync : Inport  Port=2
  Concat : xbsIndex_r4/Concat  num_inputs=8  dbl_ovrd=off  block_type=concat
      settings: block_version=9.1.01
  Concat{1..8} (8 alike) : xbsIndex_r4/Concat  num_inputs=2  dbl_ovrd=off  block_type=concat
  Constant{,3,12,17} (4 alike) : xbsIndex_r4/Constant  const=0  gui_display_data_type=Fixed-point  arith_type=Unsigned  n_bits=1  bin_pt=0  preci_type=Single  exp_width=8  frac_width=24  explicit_period=on  period=1  equ=P=C  opselect=C  inp2=PCIN>>17  opr=+  inp1=P  carry=CIN  dbl_ovrd=off  block_type=constant
  Constant{1,4,13,18} (4 alike) : xbsIndex_r4/Constant  const=1  gui_display_data_type=Fixed-point  arith_type=Unsigned  n_bits=1  bin_pt=0  preci_type=Single  exp_width=8  frac_width=24  explicit_period=on  period=1  equ=P=C  opselect=C  inp2=PCIN>>17  opr=+  inp1=P  carry=CIN  dbl_ovrd=off  block_type=constant
  Constant{2,6,8,10,14,16,20,22} (8 alike) : xbsIndex_r4/Constant  const=0  gui_display_data_type=Fixed-point  arith_type=Signed (2's comp)  n_bits=18  bin_pt=17  preci_type=Single  exp_width=8  frac_width=24  explicit_period=on  period=1  equ=P=C  opselect=C  inp2=PCIN>>17  opr=+  inp1=P  carry=CIN  dbl_ovrd=off  block_type=constant
  Constant{5,7,9,11,15,19,21,23} (8 alike) : xbsIndex_r4/Constant  const=0  gui_display_data_type=Boolean  arith_type=Boolean  n_bits=18  bin_pt=17  preci_type=Single  exp_width=8  frac_width=24  explicit_period=on  period=1  equ=P=C  opselect=C  inp2=PCIN>>17  opr=+  inp1=P  carry=CIN  dbl_ovrd=off  block_type=constant
  Counter : xbsIndex_r4/Counter  cnt_type=Free Running  cnt_to=Inf  operation=Up  start_count=0  cnt_by_val=1  arith_type=Unsigned  n_bits=9  bin_pt=0  load_pin=off  rst=on  en=off  explicit_period=on  period=1  use_behavioral_HDL=off  implementation=Fabric  use_rpm=on  dbl_ovrd=off  block_type=counter
      settings: xl_area=[0, 0, 0, 0, 0, 0, 0]  block_version=9.1.01
  Delay : xbsIndex_r4/Delay  rst=off  infoeditControl=Selection of Reset will increase slice count due to use of real FFs and instead of SRLs  en=off  latency=bram_latency+fan_latency  reg_retiming=off  dbl_ovrd=off  block_type=delay
      settings: xl_area=[0, 0, 0, 0, 0, 0, 0]  block_version=9.1.01
  Delay1 : xbsIndex_r4/Delay  rst=off  infoeditControl=Selection of Reset will increase slice count due to use of real FFs and instead of SRLs  en=off  latency=bram_latency+fan_latency  reg_retiming=off  dbl_ovrd=off  block_type=delay
      settings: xl_area=[0, 0, 0, 0, 0, 0, 0]  block_version=9.1.01
  Reinterpret{1..8} (8 alike) : xbsIndex_r4/Reinterpret  force_arith_type=on  arith_type=Unsigned  force_bin_pt=on  bin_pt=0  block_type=reinterpret
      Reinterpret1 settings: infoedit="Changes signal type without altering the binary representation.   You can changed the signal between signed and unsigned, and relocate the binary point.<P><P>Hardware notes: In hardware this block costs nothing.<P><P>Example:  Suppose the input is 6 bits wide, signed, with 2 fractional bits, and the output is forced to unsigned with 0 fractional bits.  Then an input of -2.0 (1110.00 in binary 2's complement) becomes an output of 56 (111000 in binary)."  block_version=9.1.01
      Reinterpret2 settings: infoedit="Changes signal type without altering the binary representation.   You can changed the signal between signed and unsigned, and relocate the binary point.<P><P>Hardware notes: In hardware this block costs nothing.<P><P>Example:  Suppose the input is 6 bits wide, signed, with 2 fractional bits, and the output is forced to unsigned with 0 fractional bits.  Then an input of -2.0 (1110.00 in binary 2's complement) becomes an output of 56 (111000 in binary)."  block_version=9.1.01
  c0 : xbsIndex_r4/Dual Port RAM  depth=1024  initVector=[pfb_coeff_gen_calc(12, 8,'dpss',n_inputs, nput,1,1,false), pfb_coeff_gen_calc(12, 8,'dpss',n_inputs, nput,1,2,false)]  distributed_mem=Block RAM  init_a=0  init_b=0  rst_a=off  rst_b=off  en_a=off  en_b=off  latency=1  write_mode_A=Read After Write  write_mode_B=Read After Write  optimize=Area  dbl_ovrd=off  simulation_type=fast_sim  block_type=dpram
  c1 : xbsIndex_r4/Dual Port RAM  depth=1024  initVector=[pfb_coeff_gen_calc(12, 8,'dpss',n_inputs, nput,1,3,false), pfb_coeff_gen_calc(12, 8,'dpss',n_inputs, nput,1,4,false)]  distributed_mem=Block RAM  init_a=0  init_b=0  rst_a=off  rst_b=off  en_a=off  en_b=off  latency=1  write_mode_A=Read After Write  write_mode_B=Read After Write  optimize=Area  dbl_ovrd=off  simulation_type=fast_sim  block_type=dpram
  c2 : xbsIndex_r4/Dual Port RAM  depth=1024  initVector=[pfb_coeff_gen_calc(12, 8,'dpss',n_inputs, nput,1,5,false), pfb_coeff_gen_calc(12, 8,'dpss',n_inputs, nput,1,6,false)]  distributed_mem=Block RAM  init_a=0  init_b=0  rst_a=off  rst_b=off  en_a=off  en_b=off  latency=1  write_mode_A=Read After Write  write_mode_B=Read After Write  optimize=Area  dbl_ovrd=off  simulation_type=fast_sim  block_type=dpram
  c3 : xbsIndex_r4/Dual Port RAM  depth=1024  initVector=[pfb_coeff_gen_calc(12, 8,'dpss',n_inputs, nput,1,7,false), pfb_coeff_gen_calc(12, 8,'dpss',n_inputs, nput,1,8,false)]  distributed_mem=Block RAM  init_a=0  init_b=0  rst_a=off  rst_b=off  en_a=off  en_b=off  latency=1  write_mode_A=Read After Write  write_mode_B=Read After Write  optimize=Area  dbl_ovrd=off  simulation_type=fast_sim  block_type=dpram
  fan_delay{1..8} (8 alike) : xbsIndex_r4/Delay  rst=off  infoeditControl=Selection of Reset will increase slice count due to use of real FFs and instead of SRLs  en=off  latency=fan_latency  reg_retiming=off  dbl_ovrd=off  block_type=delay
      fan_delay1 settings: block_version=11.4
      fan_delay2 settings: block_version=11.4
  dout : Outport  VectorParamsAs1DForOutWhenUnconnected=off
  sync_out : Outport  Port=2  VectorParamsAs1DForOutWhenUnconnected=off
  coeff : Outport  Port=3  VectorParamsAs1DForOutWhenUnconnected=off
  wires:
  c3/2 -> Reinterpret8/1
  c3/1 -> Reinterpret7/1
  c2/2 -> Reinterpret6/1
  c2/1 -> Reinterpret5/1
  c1/1 -> Reinterpret3/1
  fan_delay5/1 -> Concat5/2
  fan_delay6/1 -> Concat6/2
  Constant12/1 -> Concat5/1
  Constant13/1 -> Concat6/1
  Concat5/1 -> c2/1
  Constant16/1 -> c2/2
  Constant19/1 -> c2/3
  Constant21/1 -> c2/6
  Constant20/1 -> c2/5
  Concat6/1 -> c2/4
  Concat8/1 -> c3/4
  Constant14/1 -> c3/5
  Constant15/1 -> c3/6
  Constant23/1 -> c3/3
  Constant22/1 -> c3/2
  Concat7/1 -> c3/1
  Constant18/1 -> Concat8/1
  Constant17/1 -> Concat7/1
  fan_delay8/1 -> Concat8/2
  fan_delay7/1 -> Concat7/2
  fan_delay3/1 -> Concat3/2
  fan_delay4/1 -> Concat4/2
  Constant3/1 -> Concat3/1
  Constant4/1 -> Concat4/1
  Concat3/1 -> c1/1
  Constant8/1 -> c1/2
  Constant9/1 -> c1/3
  Constant11/1 -> c1/6
  Constant10/1 -> c1/5
  Concat4/1 -> c1/4
  c1/2 -> Reinterpret4/1
  c0/1 -> Reinterpret1/1
  c0/2 -> Reinterpret2/1
  Concat2/1 -> c0/4
  Constant6/1 -> c0/5
  Constant7/1 -> c0/6
  Constant5/1 -> c0/3
  Constant2/1 -> c0/2
  Concat1/1 -> c0/1
  Constant1/1 -> Concat2/1
  Constant/1 -> Concat1/1
  Reinterpret8/1 -> Concat/8
  Reinterpret7/1 -> Concat/7
  Reinterpret6/1 -> Concat/6
  Reinterpret5/1 -> Concat/5
  Reinterpret4/1 -> Concat/4
  Reinterpret3/1 -> Concat/3
  Reinterpret2/1 -> Concat/2
  fan_delay2/1 -> Concat2/2
  Reinterpret1/1 -> Concat/1
  fan_delay1/1 -> Concat1/2
  Counter/1 -> fan_delay8/1, fan_delay7/1, fan_delay6/1, fan_delay5/1, fan_delay4/1, fan_delay3/1, fan_delay2/1, fan_delay1/1
  Concat/1 -> coeff/1
  Delay/1 -> sync_out/1
  sync/1 -> Delay/1, Counter/1
  Delay1/1 -> dout/1
  din/1 -> Delay1/1
